simulink model "MX_Gateway"  (Simulink 9.0)
settings: Solver=FixedStepDiscrete  StartTime=0.0  StopTime=100  FixedStep=0.01  RelTol=1e-3
values from neighbouring files (symbols this model uses):
  ACTIVE = Bobcat.Parameter; CreateDefine( ACTIVE                         ,1                          ,'Define' ,'defines.h' )    [defines.m]
  ACTIVE_MODE_ONE = Bobcat.Parameter; CreateDefine( ACTIVE_MODE_ONE                ,19                         ,'Define' ,'defines.h' )    [defines.m]
  AUTHENTICATED = Bobcat.Parameter; CreateDefine( AUTHENTICATED                  ,1                          ,'Define' ,'defines.h' )    [defines.m]
  AUX_EMEA_CONTROLS = Bobcat.Parameter; CreateDefine( AUX_EMEA_CONTROLS  ,1   ,'Define' ,'defines.h' )    [enums.m]
  AUX_FLOW_HIGH = Bobcat.Parameter; CreateDefine( AUX_FLOW_HIGH           ,4  ,'Define' ,'defines.h' )    [enums.m]
  AUX_FLOW_INACTIVE = Bobcat.Parameter; CreateDefine( AUX_FLOW_INACTIVE       ,0  ,'Define' ,'defines.h' )    [enums.m]
  AUX_FLOW_LOW = Bobcat.Parameter; CreateDefine( AUX_FLOW_LOW            ,1  ,'Define' ,'defines.h' )    [enums.m]
  AUX_FLOW_MEDIUM = Bobcat.Parameter; CreateDefine( AUX_FLOW_MEDIUM         ,2  ,'Define' ,'defines.h' )    [enums.m]
  AUX_FLOW_STANDARD = Bobcat.Parameter; CreateDefine( AUX_FLOW_STANDARD       ,3  ,'Define' ,'defines.h' )    [enums.m]
  AUX_NON_FUNCTIONAL = Bobcat.Parameter; CreateDefine( AUX_NON_FUNCTIONAL ,255 ,'Define' ,'defines.h' )    [enums.m]
  BASE_ACTIVE = Bobcat.Parameter; CreateDefine( BASE_ACTIVE                    ,253                        ,'Define' ,'defines.h' )    [defines.m]
  BASE_DETENT = Bobcat.Parameter; CreateDefine( BASE_DETENT                    ,1                          ,'Define' ,'defines.h' )    [defines.m]
  BEEP_NONE = Bobcat.Parameter; CreateDefine( BEEP_NONE                      ,255                        ,'Define' ,'defines.h' )    [defines.m]
  CD_AIR_FILTER_DEBOUNCE_NORMAL = Bobcat.Parameter; CreateAliasCD( CD_AIR_FILTER_DEBOUNCE_NORMAL                        ,uint16(500)                ,'ConstantDataPointer' ,'CONSTANT_DATA' ,'AIR_FILTER_DEBOUNCE_NORMAL')    [cdata.m]    (also in hc12_cdata.m; m4_cdata.m)
  CD_AIR_FILTER_DEBOUNCE_NOT_CONNECTED = Bobcat.Parameter; CreateAliasCD( CD_AIR_FILTER_DEBOUNCE_NOT_CONNECTED                 ,uint16(3000)               ,'ConstantDataPointer' ,'CONSTANT_DATA' ,'AIR_FILTER_DEBOUNCE_NOT_CONNECTED')    [cdata.m]    (also in hc12_cdata.m; m4_cdata.m)
  CD_AIR_FILTER_DEBOUNCE_PLUGGED = Bobcat.Parameter; CreateAliasCD( CD_AIR_FILTER_DEBOUNCE_PLUGGED                       ,uint16(3000)               ,'ConstantDataPointer' ,'CONSTANT_DATA' ,'AIR_FILTER_DEBOUNCE_PLUGGED')    [cdata.m]    (also in hc12_cdata.m; m4_cdata.m)
  CD_AIR_FILTER_FUNCTION = Bobcat.Parameter; CreateAliasCD( CD_AIR_FILTER_FUNCTION                               ,uint8(255)                 ,'ConstantDataPointer' ,'CONSTANT_DATA' ,'AIR_FILTER_FUNCTION')    [cdata.m]    (also in hc12_cdata.m; m4_cdata.m)
  CD_AIR_FILTER_PLUGGED_WEIGHT = Bobcat.Parameter; CreateAliasCD( CD_AIR_FILTER_PLUGGED_WEIGHT                         ,uint8(2)                   ,'ConstantDataPointer' ,'CONSTANT_DATA' ,'AIR_FILTER_PLUGGED_WEIGHT')    [cdata.m]    (also in hc12_cdata.m; m4_cdata.m)
  CD_AIR_FILTER_UNPLUGGED_WEIGHT = Bobcat.Parameter; CreateAliasCD( CD_AIR_FILTER_UNPLUGGED_WEIGHT                       ,uint8(1)                   ,'ConstantDataPointer' ,'CONSTANT_DATA' ,'AIR_FILTER_UNPLUGGED_WEIGHT')    [cdata.m]    (also in hc12_cdata.m; m4_cdata.m)
  CD_AUTOIDLE_ARMBAR_DELAY = Bobcat.Parameter; CreateAliasCD( CD_AUTOIDLE_ARMBAR_DELAY                             ,uint8(2)                   ,'ConstantDataPointer' ,'CONSTANT_DATA' ,'AUTOIDLE_ARMBAR_DELAY')    [cdata.m]    (also in hc12_cdata.m; m4_cdata.m)
  CD_AUTOIDLE_FUNCTION = Bobcat.Parameter; CreateAliasCD( CD_AUTOIDLE_FUNCTION                                 ,uint8(255)                 ,'ConstantDataPointer' ,'CONSTANT_DATA' ,'AUTOIDLE_FUNCTION')    [cdata.m]    (also in hc12_cdata.m; m4_cdata.m)
  CD_AUTOIDLE_IDLE_DELAY = Bobcat.Parameter; CreateAliasCD( CD_AUTOIDLE_IDLE_DELAY                               ,uint8(40)                  ,'ConstantDataPointer' ,'CONSTANT_DATA' ,'AUTOIDLE_IDLE_DELAY')    [cdata.m]    (also in hc12_cdata.m; m4_cdata.m)
  CD_AUTOIDLE_LOW_IDLE_START = Bobcat.Parameter; CreateAliasCD( CD_AUTOIDLE_LOW_IDLE_START                           ,uint8(255)                 ,'ConstantDataPointer' ,'CONSTANT_DATA' ,'AUTOIDLE_LOW_IDLE_START')    [cdata.m]    (also in hc12_cdata.m; m4_cdata.m)
  CD_AUTOIDLE_PRESSURE_OFF = Bobcat.Parameter; CreateAliasCD( CD_AUTOIDLE_PRESSURE_OFF                             ,uint16(300)                ,'ConstantDataPointer' ,'CONSTANT_DATA' ,'AUTOIDLE_PRESSURE_OFF')    [cdata.m]    (also in hc12_cdata.m; m4_cdata.m)
  CD_AUTOIDLE_PRESSURE_ON = Bobcat.Parameter; CreateAliasCD( CD_AUTOIDLE_PRESSURE_ON                              ,uint16(270)                ,'ConstantDataPointer' ,'CONSTANT_DATA' ,'AUTOIDLE_PRESSURE_ON')    [cdata.m]    (also in hc12_cdata.m; m4_cdata.m)
  CD_AUTOSHIFT_FUNCTION = Bobcat.Parameter; CreateAliasCD( CD_AUTOSHIFT_FUNCTION                                ,uint8(255)                 ,'ConstantDataPointer' ,'CONSTANT_DATA' ,'AUTOSHIFT_FUNCTION')    [cdata.m]    (also in hc12_cdata.m; m4_cdata.m)
  CD_AUTOSHIFT_PHIGH1 = Bobcat.Parameter; CreateAliasCD( CD_AUTOSHIFT_PHIGH1                                  ,uint16(205)                ,'ConstantDataPointer' ,'CONSTANT_DATA' ,'AUTOSHIFT_PHIGH1')    [cdata.m]    (also in hc12_cdata.m; m4_cdata.m)
  CD_AUTOSHIFT_PHIGH2 = Bobcat.Parameter; CreateAliasCD( CD_AUTOSHIFT_PHIGH2                                  ,uint16(193)                ,'ConstantDataPointer' ,'CONSTANT_DATA' ,'AUTOSHIFT_PHIGH2')    [cdata.m]    (also in hc12_cdata.m; m4_cdata.m)
  CD_AUTOSHIFT_PHIGH_TEMP1 = Bobcat.Parameter; CreateAliasCD( CD_AUTOSHIFT_PHIGH_TEMP1                             ,int16(70)                  ,'ConstantDataPointer' ,'CONSTANT_DATA' ,'AUTOSHIFT_PHIGH_TEMP1')    [cdata.m]    (also in hc12_cdata.m; m4_cdata.m)
  CD_AUTOSHIFT_PHIGH_TEMP2 = Bobcat.Parameter; CreateAliasCD( CD_AUTOSHIFT_PHIGH_TEMP2                             ,int16(160)                 ,'ConstantDataPointer' ,'CONSTANT_DATA' ,'AUTOSHIFT_PHIGH_TEMP2')    [cdata.m]    (also in hc12_cdata.m; m4_cdata.m)
  CD_AUTOSHIFT_PLOW1 = Bobcat.Parameter; CreateAliasCD( CD_AUTOSHIFT_PLOW1                                   ,uint16(130)                ,'ConstantDataPointer' ,'CONSTANT_DATA' ,'AUTOSHIFT_PLOW1')    [cdata.m]    (also in hc12_cdata.m; m4_cdata.m)
  CD_AUTOSHIFT_PLOW2 = Bobcat.Parameter; CreateAliasCD( CD_AUTOSHIFT_PLOW2                                   ,uint16(100)                ,'ConstantDataPointer' ,'CONSTANT_DATA' ,'AUTOSHIFT_PLOW2')    [cdata.m]    (also in hc12_cdata.m; m4_cdata.m)
  CD_AUTOSHIFT_PLOW_TEMP1 = Bobcat.Parameter; CreateAliasCD( CD_AUTOSHIFT_PLOW_TEMP1                              ,int16(20)                  ,'ConstantDataPointer' ,'CONSTANT_DATA' ,'AUTOSHIFT_PLOW_TEMP1')    [cdata.m]    (also in hc12_cdata.m; m4_cdata.m)
  CD_AUTOSHIFT_PLOW_TEMP2 = Bobcat.Parameter; CreateAliasCD( CD_AUTOSHIFT_PLOW_TEMP2                              ,int16(80)                  ,'ConstantDataPointer' ,'CONSTANT_DATA' ,'AUTOSHIFT_PLOW_TEMP2')    [cdata.m]    (also in hc12_cdata.m; m4_cdata.m)
  CD_AUTOSHIFT_TRAVEL_DELAY = Bobcat.Parameter; CreateAliasCD( CD_AUTOSHIFT_TRAVEL_DELAY                            ,uint8(32)                  ,'ConstantDataPointer' ,'CONSTANT_DATA' ,'AUTOSHIFT_TRAVEL_DELAY')    [cdata.m]    (also in hc12_cdata.m; m4_cdata.m)
  CD_AUX2_CRACKPOINT_CURRENT = Bobcat.Parameter; CreateAliasCD( CD_AUX2_CRACKPOINT_CURRENT                           ,fi(0,0,16,12,'hex','0c28') ,'ConstantDataPointer' ,'CONSTANT_DATA' ,'AUX2_CRACKPOINT_CURRENT')    [cdata.m]    (also in hc12_cdata.m; m4_cdata.m)
  CD_AUX2_FLOW_LOW_FUNCTION = Bobcat.Parameter; CreateAliasCD( CD_AUX2_FLOW_LOW_FUNCTION                            ,uint8(255)                 ,'ConstantDataPointer' ,'CONSTANT_DATA' ,'AUX2_FLOW_LOW_FUNCTION')    [cdata.m]    (also in hc12_cdata.m; m4_cdata.m)
  CD_AUX2_FLOW_MED_FUNCTION = Bobcat.Parameter; CreateAliasCD( CD_AUX2_FLOW_MED_FUNCTION                            ,uint8(255)                 ,'ConstantDataPointer' ,'CONSTANT_DATA' ,'AUX2_FLOW_MED_FUNCTION')    [cdata.m]    (also in hc12_cdata.m; m4_cdata.m)
  CD_AUX2_HI_FLOW_PERCENT = Bobcat.Parameter; CreateAliasCD( CD_AUX2_HI_FLOW_PERCENT                              ,fi(0,0,16,16,'hex','ffff') ,'ConstantDataPointer' ,'CONSTANT_DATA' ,'AUX2_HI_FLOW_PERCENT')    [cdata.m]    (also in hc12_cdata.m; m4_cdata.m)
  CD_AUX2_LOW_FLOW_PERCENT = Bobcat.Parameter; CreateAliasCD( CD_AUX2_LOW_FLOW_PERCENT                             ,fi(0,0,16,16,'hex','7333') ,'ConstantDataPointer' ,'CONSTANT_DATA' ,'AUX2_LOW_FLOW_PERCENT')    [cdata.m]    (also in hc12_cdata.m; m4_cdata.m)
  CD_AUX2_MAX_FLOW_CURRENT = Bobcat.Parameter; CreateAliasCD( CD_AUX2_MAX_FLOW_CURRENT                             ,fi(0,0,16,12,'hex','14cc') ,'ConstantDataPointer' ,'CONSTANT_DATA' ,'AUX2_MAX_FLOW_CURRENT')    [cdata.m]    (also in hc12_cdata.m; m4_cdata.m)
  CD_AUX2_MED_FLOW_PERCENT = Bobcat.Parameter; CreateAliasCD( CD_AUX2_MED_FLOW_PERCENT                             ,fi(0,0,16,16,'hex','9999') ,'ConstantDataPointer' ,'CONSTANT_DATA' ,'AUX2_MED_FLOW_PERCENT')    [cdata.m]    (also in hc12_cdata.m; m4_cdata.m)
  CD_AUX_CRACKPOINT_CURRENT = Bobcat.Parameter; CreateAliasCD( CD_AUX_CRACKPOINT_CURRENT                            ,fi(0,0,16,12,'hex','0000') ,'ConstantDataPointer' ,'CONSTANT_DATA' ,'AUX_CRACKPOINT_CURRENT')    [cdata.m]    (also in hc12_cdata.m; m4_cdata.m)
  CD_AUX_DETENT_FLASH_TIME = Bobcat.Parameter; CreateAliasCD( CD_AUX_DETENT_FLASH_TIME                             ,uint16(72)                 ,'ConstantDataPointer' ,'CONSTANT_DATA' ,'AUX_DETENT_FLASH_TIME')    [cdata.m]    (also in hc12_cdata.m; m4_cdata.m)
  CD_AUX_DETENT_HOLD_TIME = Bobcat.Parameter; CreateAliasCD( CD_AUX_DETENT_HOLD_TIME                              ,uint16(100)                ,'ConstantDataPointer' ,'CONSTANT_DATA' ,'AUX_DETENT_HOLD_TIME')    [cdata.m]    (also in hc12_cdata.m; m4_cdata.m)
  CD_AUX_FULL_ON_AUX1 = Bobcat.Parameter; CreateAliasCD( CD_AUX_FULL_ON_AUX1                                  ,fi(0,0,16,12,'hex','10cd') ,'ConstantDataPointer' ,'CONSTANT_DATA' ,'AUX_FULL_ON_AUX1')    [cdata.m]    (also in hc12_cdata.m; m4_cdata.m)
  CD_AUX_FULL_ON_AUX2 = Bobcat.Parameter; CreateAliasCD( CD_AUX_FULL_ON_AUX2                                  ,fi(0,0,16,12,'hex','139d') ,'ConstantDataPointer' ,'CONSTANT_DATA' ,'AUX_FULL_ON_AUX2')    [cdata.m]    (also in hc12_cdata.m; m4_cdata.m)
  CD_AUX_FULL_ON_AUX3 = Bobcat.Parameter; CreateAliasCD( CD_AUX_FULL_ON_AUX3                                  ,fi(0,0,16,12,'hex','17d7') ,'ConstantDataPointer' ,'CONSTANT_DATA' ,'AUX_FULL_ON_AUX3')    [cdata.m]    (also in hc12_cdata.m; m4_cdata.m)
  CD_AUX_FUNCTION = Bobcat.Parameter; CreateAliasCD( CD_AUX_FUNCTION                                      ,uint8(0)                   ,'ConstantDataPointer' ,'CONSTANT_DATA' ,'AUX_FUNCTION')    [cdata.m]    (also in hc12_cdata.m; m4_cdata.m)
  CD_AUX_RATE_LIMIT_CRACKPOINT = Bobcat.Parameter; CreateAliasCD( CD_AUX_RATE_LIMIT_CRACKPOINT                         ,fi(0,0,16,12,'hex','0800') ,'ConstantDataPointer' ,'CONSTANT_DATA' ,'AUX_RATE_LIMIT_CRACKPOINT')    [cdata.m]    (also in hc12_cdata.m; m4_cdata.m)
  CD_AUX_RATE_LIMIT_CRACKPOINT_RATE = Bobcat.Parameter; CreateAliasCD( CD_AUX_RATE_LIMIT_CRACKPOINT_RATE                    ,fi(0,1,32,12,'int',102400) ,'ConstantDataPointer' ,'CONSTANT_DATA' ,'AUX_RATE_LIMIT_CRACKPOINT_RATE')    [cdata.m]    (also in hc12_cdata.m; m4_cdata.m)
  CD_AUX_RATE_LIMIT_FUNCTION = Bobcat.Parameter; CreateAliasCD( CD_AUX_RATE_LIMIT_FUNCTION                           ,uint8(255)                 ,'ConstantDataPointer' ,'CONSTANT_DATA' ,'AUX_RATE_LIMIT_FUNCTION')    [cdata.m]    (also in hc12_cdata.m; m4_cdata.m)
  CD_AUX_RATE_LIMIT_MAX_ENG_SPD = Bobcat.Parameter; CreateAliasCD( CD_AUX_RATE_LIMIT_MAX_ENG_SPD                        ,uint16(1600)               ,'ConstantDataPointer' ,'CONSTANT_DATA' ,'AUX_RATE_LIMIT_MAX_ENG_SPD')    [cdata.m]    (also in hc12_cdata.m; m4_cdata.m)
  CD_AUX_RATE_LIMIT_MIN_ENG_SPD = Bobcat.Parameter; CreateAliasCD( CD_AUX_RATE_LIMIT_MIN_ENG_SPD                        ,uint16(100)                ,'ConstantDataPointer' ,'CONSTANT_DATA' ,'AUX_RATE_LIMIT_MIN_ENG_SPD')    [cdata.m]    (also in hc12_cdata.m; m4_cdata.m)
  CD_AUX_RATE_LIMIT_NORMAL_RATE = Bobcat.Parameter; CreateAliasCD( CD_AUX_RATE_LIMIT_NORMAL_RATE                        ,fi(0,1,32,12,'int',2048)   ,'ConstantDataPointer' ,'CONSTANT_DATA' ,'AUX_RATE_LIMIT_NORMAL_RATE')    [cdata.m]    (also in hc12_cdata.m; m4_cdata.m)
  CD_AUXPOT_CAL_DEADBAND_HIGH = Bobcat.Parameter; CreateAliasCD( CD_AUXPOT_CAL_DEADBAND_HIGH                          ,uint16(574)                ,'ConstantDataPointer' ,'CONSTANT_DATA' ,'AUXPOT_CAL_DEADBAND_HIGH')    [cdata.m]    (also in hc12_cdata.m; m4_cdata.m)
  CD_AUXPOT_CAL_DEADBAND_LOW = Bobcat.Parameter; CreateAliasCD( CD_AUXPOT_CAL_DEADBAND_LOW                           ,uint16(424)                ,'ConstantDataPointer' ,'CONSTANT_DATA' ,'AUXPOT_CAL_DEADBAND_LOW')    [cdata.m]    (also in hc12_cdata.m; m4_cdata.m)
  CD_AUXPOT_CENTER_RATIO = Bobcat.Parameter; CreateAliasCD( CD_AUXPOT_CENTER_RATIO                               ,uint16(500)                ,'ConstantDataPointer' ,'CONSTANT_DATA' ,'AUXPOT_CENTER_RATIO')    [cdata.m]    (also in hc12_cdata.m; m4_cdata.m)
  CD_AUXPOT_DEADBAND = Bobcat.Parameter; CreateAliasCD( CD_AUXPOT_DEADBAND                                   ,uint8(38)                  ,'ConstantDataPointer' ,'CONSTANT_DATA' ,'AUXPOT_DEADBAND')    [cdata.m]    (also in hc12_cdata.m; m4_cdata.m)
  CD_AUXPOT_FUNCTION = Bobcat.Parameter; CreateAliasCD( CD_AUXPOT_FUNCTION                                   ,uint8(0)                   ,'ConstantDataPointer' ,'CONSTANT_DATA' ,'AUXPOT_FUNCTION')    [cdata.m]    (also in hc12_cdata.m; m4_cdata.m)
  CD_AUXPOT_RATIO_GAIN = Bobcat.Parameter; CreateAliasCD( CD_AUXPOT_RATIO_GAIN                                 ,uint16(1000)               ,'ConstantDataPointer' ,'CONSTANT_DATA' ,'AUXPOT_RATIO_GAIN')    [cdata.m]    (also in hc12_cdata.m; m4_cdata.m)
  CD_CAN_THROTTLE_FUNCTION = Bobcat.Parameter; CreateAliasCD( CD_CAN_THROTTLE_FUNCTION                             ,uint8(255)                 ,'ConstantDataPointer' ,'CONSTANT_DATA' ,'CAN_THROTTLE_FUNCTION')    [cdata.m]    (also in hc12_cdata.m; m4_cdata.m)
  CD_CAN_THROTTLE_REVERSE = Bobcat.Parameter; CreateAliasCD( CD_CAN_THROTTLE_REVERSE                              ,uint8(255)                 ,'ConstantDataPointer' ,'CONSTANT_DATA' ,'CAN_THROTTLE_REVERSE')    [cdata.m]    (also in hc12_cdata.m; m4_cdata.m)
  CD_CONSOLE_DEBOUNCE_ABSENT = Bobcat.Parameter; CreateAliasCD( CD_CONSOLE_DEBOUNCE_ABSENT                           ,uint16(12)                 ,'ConstantDataPointer' ,'CONSTANT_DATA' ,'CONSOLE_DEBOUNCE_ABSENT')    [cdata.m]    (also in hc12_cdata.m; m4_cdata.m)
  CD_CONSOLE_DEBOUNCE_OORH = Bobcat.Parameter; CreateAliasCD( CD_CONSOLE_DEBOUNCE_OORH                             ,uint16(12)                 ,'ConstantDataPointer' ,'CONSTANT_DATA' ,'CONSOLE_DEBOUNCE_OORH')    [cdata.m]    (also in hc12_cdata.m; m4_cdata.m)
  CD_CONSOLE_DEBOUNCE_OORL = Bobcat.Parameter; CreateAliasCD( CD_CONSOLE_DEBOUNCE_OORL                             ,uint16(12)                 ,'ConstantDataPointer' ,'CONSTANT_DATA' ,'CONSOLE_DEBOUNCE_OORL')    [cdata.m]    (also in hc12_cdata.m; m4_cdata.m)
  CD_CONSOLE_DEBOUNCE_PRESENT = Bobcat.Parameter; CreateAliasCD( CD_CONSOLE_DEBOUNCE_PRESENT                          ,uint16(12)                 ,'ConstantDataPointer' ,'CONSTANT_DATA' ,'CONSOLE_DEBOUNCE_PRESENT')    [cdata.m]    (also in hc12_cdata.m; m4_cdata.m)
  CD_CONSOLE_FUNCTION = Bobcat.Parameter; CreateAliasCD( CD_CONSOLE_FUNCTION                                  ,uint8(0)                   ,'ConstantDataPointer' ,'CONSTANT_DATA' ,'CONSOLE_FUNCTION')    [cdata.m]    (also in hc12_cdata.m; m4_cdata.m)
  CD_CONSOLE_OORH = Bobcat.Parameter; CreateAliasCD( CD_CONSOLE_OORH                                      ,uint16(740)                ,'ConstantDataPointer' ,'CONSTANT_DATA' ,'CONSOLE_OORH')    [cdata.m]    (also in hc12_cdata.m; m4_cdata.m)
  CD_CONSOLE_OORL = Bobcat.Parameter; CreateAliasCD( CD_CONSOLE_OORL                                      ,uint16(10)                 ,'ConstantDataPointer' ,'CONSTANT_DATA' ,'CONSOLE_OORL')    [cdata.m]    (also in hc12_cdata.m; m4_cdata.m)
  CD_CONSOLE_PRESENT_HIGH = Bobcat.Parameter; CreateAliasCD( CD_CONSOLE_PRESENT_HIGH                              ,uint16(515)                ,'ConstantDataPointer' ,'CONSTANT_DATA' ,'CONSOLE_PRESENT_HIGH')    [cdata.m]    (also in hc12_cdata.m; m4_cdata.m)
  CD_CONSOLE_PRESENT_LOW = Bobcat.Parameter; CreateAliasCD( CD_CONSOLE_PRESENT_LOW                               ,uint16(375)                ,'ConstantDataPointer' ,'CONSTANT_DATA' ,'CONSOLE_PRESENT_LOW')    [cdata.m]    (also in hc12_cdata.m; m4_cdata.m)
  CD_DELUXE_GEN5_5_FUNCTION = Bobcat.Parameter; CreateAliasCD( CD_DELUXE_GEN5_5_FUNCTION                            ,uint8(255)                 ,'ConstantDataPointer' ,'CONSTANT_DATA' ,'DELUXE_GEN5_5_FUNCTION')    [cdata.m]    (also in hc12_cdata.m; m4_cdata.m)
  CD_DELUXE_GEN5_FUNCTION = Bobcat.Parameter; CreateAliasCD( CD_DELUXE_GEN5_FUNCTION                              ,uint8(255)                 ,'ConstantDataPointer' ,'CONSTANT_DATA' ,'DELUXE_GEN5_FUNCTION')    [cdata.m]    (also in hc12_cdata.m; m4_cdata.m)
  CD_DERATE_ENGINE_SPEED_DEBOUNCE_HIGH = Bobcat.Parameter; CreateAliasCD( CD_DERATE_ENGINE_SPEED_DEBOUNCE_HIGH                 ,uint16(200)                ,'ConstantDataPointer' ,'CONSTANT_DATA' ,'DERATE_ENGINE_SPEED_DEBOUNCE_HIGH')    [cdata.m]    (also in hc12_cdata.m; m4_cdata.m)
  CD_DERATE_ENGINE_SPEED_DEBOUNCE_SHUTDOWN = Bobcat.Parameter; CreateAliasCD( CD_DERATE_ENGINE_SPEED_DEBOUNCE_SHUTDOWN             ,uint16(1000)               ,'ConstantDataPointer' ,'CONSTANT_DATA' ,'DERATE_ENGINE_SPEED_DEBOUNCE_SHUTDOWN')    [cdata.m]    (also in hc12_cdata.m; m4_cdata.m)
  CD_DERATE_ENGINE_SPEED_HIGH_OFFSET = Bobcat.Parameter; CreateAliasCD( CD_DERATE_ENGINE_SPEED_HIGH_OFFSET                   ,uint16(200)                ,'ConstantDataPointer' ,'CONSTANT_DATA' ,'DERATE_ENGINE_SPEED_HIGH_OFFSET')    [cdata.m]    (also in hc12_cdata.m; m4_cdata.m)
  CD_DERATE_METHOD = Bobcat.Parameter; CreateAliasCD( CD_DERATE_METHOD                                     ,uint8(0)                   ,'ConstantDataPointer' ,'CONSTANT_DATA' ,'DERATE_METHOD')    [cdata.m]    (also in hc12_cdata.m; m4_cdata.m)
  CD_DUMP_VALVE_FUNCTION = Bobcat.Parameter; CreateAliasCD( CD_DUMP_VALVE_FUNCTION                               ,uint8(255)                 ,'ConstantDataPointer' ,'CONSTANT_DATA' ,'DUMP_VALVE_FUNCTION')    [cdata.m]    (also in hc12_cdata.m; m4_cdata.m)
  CD_DUMP_VALVE_ON_CURRENT = Bobcat.Parameter; CreateAliasCD( CD_DUMP_VALVE_ON_CURRENT                             ,fi(0,0,16,12,'hex','ffff') ,'ConstantDataPointer' ,'CONSTANT_DATA' ,'DUMP_VALVE_ON_CURRENT')    [cdata.m]    (also in hc12_cdata.m; m4_cdata.m)
  CD_ECO_MODE_THROTTLE_REDUCTION = Bobcat.Parameter; CreateAliasCD( CD_ECO_MODE_THROTTLE_REDUCTION                       ,uint16(850)                ,'ConstantDataPointer' ,'CONSTANT_DATA' ,'ECO_MODE_THROTTLE_REDUCTION')    [cdata.m]    (also in hc12_cdata.m; m4_cdata.m)
  CD_ECT_FILTER_GAIN = Bobcat.Parameter; CreateAliasCD( CD_ECT_FILTER_GAIN                                   ,uint8(6)                   ,'ConstantDataPointer' ,'CONSTANT_DATA' ,'ECT_FILTER_GAIN')    [cdata.m]    (also in hc12_cdata.m; m4_cdata.m)
  CD_ECU_FUNCTION = Bobcat.Parameter; CreateAliasCD( CD_ECU_FUNCTION                                      ,uint8(255)                 ,'ConstantDataPointer' ,'CONSTANT_DATA' ,'ECU_FUNCTION')    [cdata.m]    (also in hc12_cdata.m; m4_cdata.m)
  CD_ECU_HOLD_DEBOUNCE_ERROR_ON = Bobcat.Parameter; CreateAliasCD( CD_ECU_HOLD_DEBOUNCE_ERROR_ON                        ,uint16(20)                 ,'ConstantDataPointer' ,'CONSTANT_DATA' ,'ECU_HOLD_DEBOUNCE_ERROR_ON')    [cdata.m]    (also in hc12_cdata.m; m4_cdata.m)
  CD_EMEA_CONTROLS_HOLD_TIME = Bobcat.Parameter; CreateAliasCD( CD_EMEA_CONTROLS_HOLD_TIME                           ,uint16(30)                 ,'ConstantDataPointer' ,'CONSTANT_DATA' ,'EMEA_CONTROLS_HOLD_TIME')    [cdata.m]    (also in hc12_cdata.m; m4_cdata.m)
  CD_EMEA_CONTROLS_OPTION_A = Bobcat.Parameter; CreateAliasCD( CD_EMEA_CONTROLS_OPTION_A                            ,uint8(255)                 ,'ConstantDataPointer' ,'CONSTANT_DATA' ,'EMEA_CONTROLS_OPTION_A')    [cdata.m]    (also in hc12_cdata.m; m4_cdata.m)
  CD_EMEA_CONTROLS_OPTION_C = Bobcat.Parameter; CreateAliasCD( CD_EMEA_CONTROLS_OPTION_C                            ,uint8(255)                 ,'ConstantDataPointer' ,'CONSTANT_DATA' ,'EMEA_CONTROLS_OPTION_C')    [cdata.m]    (also in hc12_cdata.m; m4_cdata.m)
  CD_EMEA_CONTROLS_REVERSE = Bobcat.Parameter; CreateAliasCD( CD_EMEA_CONTROLS_REVERSE                             ,uint8(255)                 ,'ConstantDataPointer' ,'CONSTANT_DATA' ,'EMEA_CONTROLS_REVERSE')    [cdata.m]    (also in hc12_cdata.m; m4_cdata.m)
  CD_EMEA_ELECTRIC_VERSION = Bobcat.Parameter; CreateAliasCD( CD_EMEA_ELECTRIC_VERSION                             ,uint8(0)                   ,'ConstantDataPointer' ,'CONSTANT_DATA' ,'EMEA_ELECTRIC_VERSION')    [cdata.m]    (also in hc12_cdata.m; m4_cdata.m)
  CD_EMEA_POT_CAL_DEADBAND_HIGH = Bobcat.Parameter; CreateAliasCD( CD_EMEA_POT_CAL_DEADBAND_HIGH                        ,uint16(574)                ,'ConstantDataPointer' ,'CONSTANT_DATA' ,'EMEA_POT_CAL_DEADBAND_HIGH')    [cdata.m]    (also in hc12_cdata.m; m4_cdata.m)
  CD_EMEA_POT_CAL_DEADBAND_LOW = Bobcat.Parameter; CreateAliasCD( CD_EMEA_POT_CAL_DEADBAND_LOW                         ,uint16(424)                ,'ConstantDataPointer' ,'CONSTANT_DATA' ,'EMEA_POT_CAL_DEADBAND_LOW')    [cdata.m]    (also in hc12_cdata.m; m4_cdata.m)
  CD_ENGINE_RPM_FACTOR = Bobcat.Parameter; CreateAliasCD( CD_ENGINE_RPM_FACTOR                                 ,uint8(35)                  ,'ConstantDataPointer' ,'CONSTANT_DATA' ,'ENGINE_RPM_FACTOR')    [cdata.m]    (also in hc12_cdata.m; m4_cdata.m)
  CD_ENGINE_SPEED_FILTER_FAST_VALUE = Bobcat.Parameter; CreateAliasCD( CD_ENGINE_SPEED_FILTER_FAST_VALUE                    ,uint8(1)                   ,'ConstantDataPointer' ,'CONSTANT_DATA' ,'ENGINE_SPEED_FILTER_FAST_VALUE')    [cdata.m]    (also in hc12_cdata.m; m4_cdata.m)
  CD_ENGINE_SPEED_FILTER_SLOW_VALUE = Bobcat.Parameter; CreateAliasCD( CD_ENGINE_SPEED_FILTER_SLOW_VALUE                    ,uint8(4)                   ,'ConstantDataPointer' ,'CONSTANT_DATA' ,'ENGINE_SPEED_FILTER_SLOW_VALUE')    [cdata.m]    (also in hc12_cdata.m; m4_cdata.m)
  CD_ENGINE_SPEED_RUNNING = Bobcat.Parameter; CreateAliasCD( CD_ENGINE_SPEED_RUNNING                              ,uint16(800)                ,'ConstantDataPointer' ,'CONSTANT_DATA' ,'ENGINE_SPEED_RUNNING')    [cdata.m]    (also in hc12_cdata.m; m4_cdata.m)
  CD_ENGINE_START_DELAY_DEBOUNCE_RPM_NO_SIGNAL = Bobcat.Parameter; CreateAliasCD( CD_ENGINE_START_DELAY_DEBOUNCE_RPM_NO_SIGNAL         ,uint16(0)                  ,'ConstantDataPointer' ,'CONSTANT_DATA' ,'ENGINE_START_DELAY_DEBOUNCE_RPM_NO_SIGNAL')    [cdata.m]    (also in hc12_cdata.m; m4_cdata.m)
  CD_ENGINE_START_DELAY_OFF_TIME = Bobcat.Parameter; CreateAliasCD( CD_ENGINE_START_DELAY_OFF_TIME                       ,uint16(0)                  ,'ConstantDataPointer' ,'CONSTANT_DATA' ,'ENGINE_START_DELAY_OFF_TIME')    [cdata.m]    (also in hc12_cdata.m; m4_cdata.m)
  CD_ENGINE_START_DELAY_RPM_NO_SIGNAL = Bobcat.Parameter; CreateAliasCD( CD_ENGINE_START_DELAY_RPM_NO_SIGNAL                  ,uint16(0)                  ,'ConstantDataPointer' ,'CONSTANT_DATA' ,'ENGINE_START_DELAY_RPM_NO_SIGNAL')    [cdata.m]    (also in hc12_cdata.m; m4_cdata.m)
  CD_EOP_DEBOUNCE_EXTREMELY_LOW = Bobcat.Parameter; CreateAliasCD( CD_EOP_DEBOUNCE_EXTREMELY_LOW                        ,uint16(50)                 ,'ConstantDataPointer' ,'CONSTANT_DATA' ,'EOP_DEBOUNCE_EXTREMELY_LOW')    [cdata.m]    (also in hc12_cdata.m; m4_cdata.m)
  CD_EOP_DEBOUNCE_NORMAL = Bobcat.Parameter; CreateAliasCD( CD_EOP_DEBOUNCE_NORMAL                               ,uint16(50)                 ,'ConstantDataPointer' ,'CONSTANT_DATA' ,'EOP_DEBOUNCE_NORMAL')    [cdata.m]    (also in hc12_cdata.m; m4_cdata.m)
  CD_EOP_DEBOUNCE_SHUTDOWN = Bobcat.Parameter; CreateAliasCD( CD_EOP_DEBOUNCE_SHUTDOWN                             ,uint16(100)                ,'ConstantDataPointer' ,'CONSTANT_DATA' ,'EOP_DEBOUNCE_SHUTDOWN')    [cdata.m]    (also in hc12_cdata.m; m4_cdata.m)
  CD_EOP_FILTER_VALUE = Bobcat.Parameter; CreateAliasCD( CD_EOP_FILTER_VALUE                                  ,uint8(3)                   ,'ConstantDataPointer' ,'CONSTANT_DATA' ,'EOP_FILTER_VALUE')    [cdata.m]    (also in hc12_cdata.m; m4_cdata.m)
  CD_EOP_FUNCTION = Bobcat.Parameter; CreateAliasCD( CD_EOP_FUNCTION                                      ,uint8(1)                   ,'ConstantDataPointer' ,'CONSTANT_DATA' ,'EOP_FUNCTION')    [cdata.m]    (also in hc12_cdata.m; m4_cdata.m)
  CD_EOP_SWITCH_GAUGE_VALUE = Bobcat.Parameter; CreateAliasCD( CD_EOP_SWITCH_GAUGE_VALUE                            ,uint16(125)                ,'ConstantDataPointer' ,'CONSTANT_DATA' ,'EOP_SWITCH_GAUGE_VALUE')    [cdata.m]    (also in hc12_cdata.m; m4_cdata.m)
  CD_EOP_SWITCH_LOW = Bobcat.Parameter; CreateAliasCD( CD_EOP_SWITCH_LOW                                    ,uint16(50)                 ,'ConstantDataPointer' ,'CONSTANT_DATA' ,'EOP_SWITCH_LOW')    [cdata.m]    (also in hc12_cdata.m; m4_cdata.m)
  CD_EOP_SWITCH_PRESSURE_VALUE = Bobcat.Parameter; CreateAliasCD( CD_EOP_SWITCH_PRESSURE_VALUE                         ,uint16(40)                 ,'ConstantDataPointer' ,'CONSTANT_DATA' ,'EOP_SWITCH_PRESSURE_VALUE')    [cdata.m]    (also in hc12_cdata.m; m4_cdata.m)
  CD_EOT_DEBOUNCE_EXTREMELY_LOW = Bobcat.Parameter; CreateAliasCD( CD_EOT_DEBOUNCE_EXTREMELY_LOW                        ,uint16(2)                  ,'ConstantDataPointer' ,'CONSTANT_DATA' ,'EOT_DEBOUNCE_EXTREMELY_LOW')    [cdata.m]    (also in hc12_cdata.m; m4_cdata.m)
  CD_EOT_DEBOUNCE_LOW = Bobcat.Parameter; CreateAliasCD( CD_EOT_DEBOUNCE_LOW                                  ,uint16(2)                  ,'ConstantDataPointer' ,'CONSTANT_DATA' ,'EOT_DEBOUNCE_LOW')    [cdata.m]    (also in hc12_cdata.m; m4_cdata.m)
  CD_EOT_DEBOUNCE_NORMAL = Bobcat.Parameter; CreateAliasCD( CD_EOT_DEBOUNCE_NORMAL                               ,uint16(2)                  ,'ConstantDataPointer' ,'CONSTANT_DATA' ,'EOT_DEBOUNCE_NORMAL')    [cdata.m]    (also in hc12_cdata.m; m4_cdata.m)
  CD_EOT_EXTREMELY_LOW = Bobcat.Parameter; CreateAliasCD( CD_EOT_EXTREMELY_LOW                                 ,uint16(81)                 ,'ConstantDataPointer' ,'CONSTANT_DATA' ,'EOT_EXTREMELY_LOW')    [cdata.m]    (also in hc12_cdata.m; m4_cdata.m)
  CD_EOT_FUNCTION = Bobcat.Parameter; CreateAliasCD( CD_EOT_FUNCTION                                      ,uint8(255)                 ,'ConstantDataPointer' ,'CONSTANT_DATA' ,'EOT_FUNCTION')    [cdata.m]    (also in hc12_cdata.m; m4_cdata.m)
  CD_EOT_LOW = Bobcat.Parameter; CreateAliasCD( CD_EOT_LOW                                           ,uint16(90)                 ,'ConstantDataPointer' ,'CONSTANT_DATA' ,'EOT_LOW')    [cdata.m]    (also in hc12_cdata.m; m4_cdata.m)
  CD_EOT_TEMPERATURE_HYSTERESIS = Bobcat.Parameter; CreateAliasCD( CD_EOT_TEMPERATURE_HYSTERESIS                        ,uint16(2)                  ,'ConstantDataPointer' ,'CONSTANT_DATA' ,'EOT_TEMPERATURE_HYSTERESIS')    [cdata.m]    (also in hc12_cdata.m; m4_cdata.m)
  CD_FAST_TRAVEL_FUNCTION = Bobcat.Parameter; CreateAliasCD( CD_FAST_TRAVEL_FUNCTION                              ,uint8(255)                 ,'ConstantDataPointer' ,'CONSTANT_DATA' ,'FAST_TRAVEL_FUNCTION')    [cdata.m]    (also in hc12_cdata.m; m4_cdata.m)
  CD_FAST_TRAVEL_THROTTLE_DELAY = Bobcat.Parameter; CreateAliasCD( CD_FAST_TRAVEL_THROTTLE_DELAY                        ,uint16(10)                 ,'ConstantDataPointer' ,'CONSTANT_DATA' ,'FAST_TRAVEL_THROTTLE_DELAY')    [cdata.m]    (also in hc12_cdata.m; m4_cdata.m)
  CD_FAST_TRAVEL_THROTTLE_LIMIT = Bobcat.Parameter; CreateAliasCD( CD_FAST_TRAVEL_THROTTLE_LIMIT                        ,uint16(1000)               ,'ConstantDataPointer' ,'CONSTANT_DATA' ,'FAST_TRAVEL_THROTTLE_LIMIT')    [cdata.m]    (also in hc12_cdata.m; m4_cdata.m)
  CD_FLYWHEEL_TEETH = Bobcat.Parameter; CreateAliasCD( CD_FLYWHEEL_TEETH                                    ,uint8(83)                  ,'ConstantDataPointer' ,'CONSTANT_DATA' ,'FLYWHEEL_TEETH')    [cdata.m]    (also in hc12_cdata.m; m4_cdata.m)
  CD_FUEL_LEVEL_FILTER_FAST_VALUE = Bobcat.Parameter; CreateAliasCD( CD_FUEL_LEVEL_FILTER_FAST_VALUE                      ,uint8(5)                   ,'ConstantDataPointer' ,'CONSTANT_DATA' ,'FUEL_LEVEL_FILTER_FAST_VALUE')    [cdata.m]    (also in hc12_cdata.m; m4_cdata.m)
  CD_FUEL_LEVEL_FILTER_SLOW_VALUE = Bobcat.Parameter; CreateAliasCD( CD_FUEL_LEVEL_FILTER_SLOW_VALUE                      ,uint8(10)                  ,'ConstantDataPointer' ,'CONSTANT_DATA' ,'FUEL_LEVEL_FILTER_SLOW_VALUE')    [cdata.m]    (also in hc12_cdata.m; m4_cdata.m)
  CD_FUEL_PRIMARY_FEEDBACK_FUNCTION = Bobcat.Parameter; CreateAliasCD( CD_FUEL_PRIMARY_FEEDBACK_FUNCTION                    ,uint8(0)                   ,'ConstantDataPointer' ,'CONSTANT_DATA' ,'FUEL_PRIMARY_FEEDBACK_FUNCTION')    [cdata.m]    (also in hc12_cdata.m; m4_cdata.m)
  ... (+246 more)
undefined symbols (not defined in the file or in its folder): sigbuilder_block
undefined symbols (not defined in the file): CD_FUEL_RESOLUTION_TIMER
libraries: simulink x186, MX_Library x107
(part 32 of 48)

== stateflow chart MX_Gtwy_Control/System/Aux_Enable/Aux_Control ==
  state Detent_State [parallel]
    settings: executionOrder=2
    state Non_Functional
      en:
      state_DetentEnable = INACTIVE;
      state_Detent = NO_DETENT;
    state EMEA_CONTROLS
      state Enable [parallel]
        settings: executionOrder=1
        state Disabled
          en:
          state_DetentEnable = INACTIVE;
        state Enabled
          en:
          state_DetentEnable = ACTIVE;
        (default) -> Detent_State.EMEA_CONTROLS.Enable.Disabled
        Detent_State.EMEA_CONTROLS.Enable.Disabled -> Detent_State.EMEA_CONTROLS.Enable.Enabled : [state_AuxEnable == ACTIVE_MODE_ONE...
            | && aux_DetentHold == ACTIVE...
            | && state_Buttons == NORMAL...
            | && mode_RightControl ==MODE_RIGHT_AUX1]
            | {state_Buzzer = SINGLE_SHORT_BEEP;}
        Detent_State.EMEA_CONTROLS.Enable.Enabled -> Detent_State.EMEA_CONTROLS.Enable.Disabled [order 1] : [state_Buttons != NORMAL...
            | || state_AuxEnable == INACTIVE...
            | || state_Console != ACTIVE]
        Detent_State.EMEA_CONTROLS.Enable.Enabled -> Detent_State.EMEA_CONTROLS.Enable.Disabled [order 2] : [aux_DetentHold == ACTIVE]
            | {send(disable_aux,Aux_Enable_State);
            | state_Buzzer = SINGLE_SHORT_BEEP;}
        Detent_State.EMEA_CONTROLS.Enable.Enabled -> Detent_State.EMEA_CONTROLS.Enable.Disabled [order 3] : disable_detent
        Detent_State.EMEA_CONTROLS.Enable.Enabled -> Detent_State.EMEA_CONTROLS.Enable.Disabled [order 4] : [mode_RightControl != MODE_RIGHT_AUX1]
      state Detent [parallel]
        settings: executionOrder=2
        state Active_Normal
          state Detent_Base
            en:
            state_Detent = BASE_DETENT;
          state Detent_Rod
            en:
            state_Detent = ROD_DETENT;
          (junction 2081) -> Detent_State.EMEA_CONTROLS.Detent.Active_Normal.Detent_Base [order 2]
          Detent_State.EMEA_CONTROLS.Detent.Active_Normal.Detent_Rod -> Detent_State.EMEA_CONTROLS.Detent.Active_Normal.Detent_Base [order 1] : [DetentRisingEdge &&...
              |  state_AuxPot ==...
              |  BASE_ACTIVE]
          Detent_State.EMEA_CONTROLS.Detent.Active_Normal.Detent_Base -> Detent_State.EMEA_CONTROLS.Detent.Active_Normal.Detent_Rod [order 1] : [DetentRisingEdge &&...
              |  state_RightPot ==...
              |  ROD_ACTIVE]
          (junction 2081) -> Detent_State.EMEA_CONTROLS.Detent.Active_Normal.Detent_Rod [order 1] : [state_RightPot ==...
              |  ROD_ACTIVE]
        state No_Detent
          en:
          state_Detent = NO_DETENT;
        state Not_Enabled
          en:
          state_Detent = ERR_DETENT;
          state_Buzzer = DOUBLE_SHORT_BEEP;
        (default) -> Detent_State.EMEA_CONTROLS.Detent.No_Detent
        Detent_State.EMEA_CONTROLS.Detent.Active_Normal.Detent_Base -> Detent_State.EMEA_CONTROLS.Detent.No_Detent [order 2] : [DetentRisingEdge]
        Detent_State.EMEA_CONTROLS.Detent.No_Detent -> Detent_State.EMEA_CONTROLS.Detent.Not_Enabled [order 2] : [DetentRisingEdge...
            |  && mode_RightControl ==MODE_RIGHT_AUX1...
            |  && state_DetentEnable == INACTIVE...
            |  && state_AuxEnable == ACTIVE_MODE_ONE...
            |  && state_RightPot != NOT_IN_NEUTRAL]
        Detent_State.EMEA_CONTROLS.Detent.Not_Enabled -> Detent_State.EMEA_CONTROLS.Detent.No_Detent : [after(CD_AUX_DETENT_FLASH_TIME * 10, tick)]
        Detent_State.EMEA_CONTROLS.Detent.No_Detent -> (junction 2081) [order 1] : [DetentRisingEdge...
            |  && mode_RightControl ==MODE_RIGHT_AUX1...
            |  && state_DetentEnable == ACTIVE...
            |  && state_AuxEnable == ACTIVE_MODE_ONE...
            |  && state_RightPot != NOT_IN_NEUTRAL ]
        Detent_State.EMEA_CONTROLS.Detent.Active_Normal.Detent_Rod -> Detent_State.EMEA_CONTROLS.Detent.No_Detent [order 2] : [DetentRisingEdge]
        Detent_State.EMEA_CONTROLS.Detent.Active_Normal -> Detent_State.EMEA_CONTROLS.Detent.No_Detent : [aux_DebounceButton...
            | || state_DetentEnable == INACTIVE...
            | || state_AuxEnable == INACTIVE]
    state NORMAL_CONTROLS
      state Enable [parallel]
        settings: executionOrder=1
        state Disabled
          en:
          state_DetentEnable = INACTIVE;
        state Enabled
          en:
          state_DetentEnable = ACTIVE;
        (default) -> Detent_State.NORMAL_CONTROLS.Enable.Disabled
        Detent_State.NORMAL_CONTROLS.Enable.Disabled -> Detent_State.NORMAL_CONTROLS.Enable.Enabled : [state_AuxEnable == ACTIVE_MODE_ONE...
            | && aux_DetentHold == ACTIVE...
            | && state_Buttons == NORMAL]
        Detent_State.NORMAL_CONTROLS.Enable.Enabled -> Detent_State.NORMAL_CONTROLS.Enable.Disabled [order 1] : [state_Buttons != NORMAL...
            | || state_AuxEnable == INACTIVE...
            | || state_Console != ACTIVE]
        Detent_State.NORMAL_CONTROLS.Enable.Enabled -> Detent_State.NORMAL_CONTROLS.Enable.Disabled [order 2] : [aux_DetentHold == ACTIVE]
            | {send(disable_aux,Aux_Enable_State);}
        Detent_State.NORMAL_CONTROLS.Enable.Enabled -> Detent_State.NORMAL_CONTROLS.Enable.Disabled [order 3] : disable_detent
      state Detent [parallel]
        settings: executionOrder=2
        state Active_Normal
          state Detent_Base
            en:
            state_Detent = BASE_DETENT;
          state Detent_Rod
            en:
            state_Detent = ROD_DETENT;
          (junction 2098) -> Detent_State.NORMAL_CONTROLS.Detent.Active_Normal.Detent_Base [order 2]
          Detent_State.NORMAL_CONTROLS.Detent.Active_Normal.Detent_Rod -> Detent_State.NORMAL_CONTROLS.Detent.Active_Normal.Detent_Base [order 1] : [DetentRisingEdge &&...
              |  state_AuxPot ==...
              |  BASE_ACTIVE]
          Detent_State.NORMAL_CONTROLS.Detent.Active_Normal.Detent_Base -> Detent_State.NORMAL_CONTROLS.Detent.Active_Normal.Detent_Rod [order 1] : [DetentRisingEdge &&...
              |  state_AuxPot ==...
              |  ROD_ACTIVE]
          (junction 2098) -> Detent_State.NORMAL_CONTROLS.Detent.Active_Normal.Detent_Rod [order 1] : [state_AuxPot ==...
              |  ROD_ACTIVE]
        state No_Detent
          en:
          state_Detent = NO_DETENT;
        state Not_Enabled
          en:
          state_Detent = ERR_DETENT;
          state_Buzzer = DOUBLE_SHORT_BEEP;
        (default) -> Detent_State.NORMAL_CONTROLS.Detent.No_Detent
        Detent_State.NORMAL_CONTROLS.Detent.Active_Normal.Detent_Base -> Detent_State.NORMAL_CONTROLS.Detent.No_Detent [order 2] : [DetentRisingEdge]
        Detent_State.NORMAL_CONTROLS.Detent.No_Detent -> Detent_State.NORMAL_CONTROLS.Detent.Not_Enabled [order 2] : [DetentRisingEdge...
            |  && state_DetentEnable == INACTIVE...
            |  && state_AuxEnable == ACTIVE_MODE_ONE...
            |  && state_AuxPot != NOT_IN_NEUTRAL]
        Detent_State.NORMAL_CONTROLS.Detent.No_Detent -> (junction 2098) [order 1] : [DetentRisingEdge...
            |  && state_DetentEnable == ACTIVE...
            |  && state_AuxEnable == ACTIVE_MODE_ONE...
            |  && state_AuxPot != NOT_IN_NEUTRAL ]
        Detent_State.NORMAL_CONTROLS.Detent.Not_Enabled -> Detent_State.NORMAL_CONTROLS.Detent.No_Detent : [after(CD_AUX_DETENT_FLASH_TIME * 10, tick)]
        Detent_State.NORMAL_CONTROLS.Detent.Active_Normal.Detent_Rod -> Detent_State.NORMAL_CONTROLS.Detent.No_Detent [order 2] : [DetentRisingEdge]
        Detent_State.NORMAL_CONTROLS.Detent.Active_Normal -> Detent_State.NORMAL_CONTROLS.Detent.No_Detent : [aux_DebounceButton...
            | || state_DetentEnable == INACTIVE...
            | || state_AuxEnable == INACTIVE]
    (default) -> Detent_State.Non_Functional
    Detent_State.Non_Functional -> (junction 2063)
    (junction 2063) -> Detent_State.EMEA_CONTROLS [order 1] : [CD_RIGHT_POT_FUNCTION !=NON_FUNCTIONAL]
    (junction 2063) -> NORMAL_CONTROLS [order 2] : [CD_AUX_FUNCTION != NON_FUNCTIONAL]
  state Aux_Enable_State [parallel]
    settings: executionOrder=1
    state Non_Functional
      en:
      state_AuxEnable = INACTIVE;
      aux_Flow = AUX_FLOW_INACTIVE;
    state PHOENIX_K2
      state Functional
        state Enabled
          state Off
            en:
            aux_Flow = AUX_FLOW_INACTIVE;
            ee_AuxFlow = AUX_FLOW_INACTIVE;
            state_AuxEnable = INACTIVE;
            matlab_UpdateEEVar();
          state Active
            state Low_Flow
              en:
              aux_Flow = AUX_FLOW_LOW;
              ee_AuxFlow = AUX_FLOW_LOW;
              state_AuxEnable = ACTIVE_MODE_ONE;
              matlab_UpdateEEVar();
            state Medium_Flow
              en:
              aux_Flow = AUX_FLOW_MEDIUM;
              ee_AuxFlow = AUX_FLOW_MEDIUM;
              state_AuxEnable = ACTIVE_MODE_ONE;
              matlab_UpdateEEVar();
            state Standard_Flow
              en:
              aux_Flow = AUX_FLOW_STANDARD;
              ee_AuxFlow = AUX_FLOW_STANDARD;
              state_AuxEnable = ACTIVE_MODE_ONE;
              matlab_UpdateEEVar();
            Aux_Enable_State.PHOENIX_K2.Functional.Enabled.Active.Medium_Flow -> Aux_Enable_State.PHOENIX_K2.Functional.Enabled.Active.Low_Flow : [aux_DebounceButton]
                | {state_Buzzer = SINGLE_SHORT_BEEP;}
            Aux_Enable_State.PHOENIX_K2.Functional.Enabled.Active.Low_Flow -> Aux_Enable_State.PHOENIX_K2.Functional.Enabled.Active.Standard_Flow [order 1] : [aux_DebounceButton...
                | && state_DetentEnable == ACTIVE]
                | {state_Buzzer = SINGLE_SHORT_BEEP;}
            (junction 2153) -> (junction 2154) [order 1] : [CD_AUX_ADJ_FLOW_FUNCTION == NON_FUNCTIONAL]
            (junction 2153) -> Aux_Enable_State.PHOENIX_K2.Functional.Enabled.Active.Standard_Flow [order 2] : {state_Buzzer = SINGLE_SHORT_BEEP;}
            (junction 2154) -> Aux_Enable_State.PHOENIX_K2.Functional.Enabled.Active.Standard_Flow
            Aux_Enable_State.PHOENIX_K2.Functional.Enabled.Active.Standard_Flow -> Aux_Enable_State.PHOENIX_K2.Functional.Enabled.Active.Medium_Flow [order 1] : [aux_DebounceButton...
                | && CD_AUX_ADJ_FLOW_FUNCTION != NON_FUNCTIONAL]
                | {state_Buzzer = SINGLE_SHORT_BEEP;}
          (default) -> (junction 2137)
          (junction 2137) -> Aux_Enable_State.PHOENIX_K2.Functional.Enabled.Off [order 1] : [ee_AuxFlow == AUX_FLOW_INACTIVE]
          (junction 2137) -> (junction 2138) [order 2]
          Aux_Enable_State.PHOENIX_K2.Functional.Enabled.Active.Low_Flow -> Aux_Enable_State.PHOENIX_K2.Functional.Enabled.Off [order 2] : [aux_DebounceButton...
              | && state_DetentEnable != ACTIVE]
              | {state_Buzzer = SINGLE_SHORT_BEEP;}
          Aux_Enable_State.PHOENIX_K2.Functional.Enabled.Off -> (junction 2153) : [aux_DebounceButton...
              | || aux_DetentHold]
          Aux_Enable_State.PHOENIX_K2.Functional.Enabled.Active.Standard_Flow -> Aux_Enable_State.PHOENIX_K2.Functional.Enabled.Off [order 2] : [aux_DebounceButton...
              | && CD_AUX_ADJ_FLOW_FUNCTION == NON_FUNCTIONAL]
          Aux_Enable_State.PHOENIX_K2.Functional.Enabled.Active -> Aux_Enable_State.PHOENIX_K2.Functional.Enabled.Off : disable_aux
          (junction 2138) -> Aux_Enable_State.PHOENIX_K2.Functional.Enabled.Active.Low_Flow [order 1] : [ee_AuxFlow == AUX_FLOW_LOW...
              | && CD_AUX_ADJ_FLOW_FUNCTION != NON_FUNCTIONAL]
          (junction 2138) -> (junction 2139) [order 2]
          (junction 2139) -> Aux_Enable_State.PHOENIX_K2.Functional.Enabled.Active.Medium_Flow [order 1] : [ee_AuxFlow == AUX_FLOW_MEDIUM...
              | && CD_AUX_ADJ_FLOW_FUNCTION != NON_FUNCTIONAL]
          (junction 2139) -> (junction 2140) [order 2]
          (junction 2140) -> Aux_Enable_State.PHOENIX_K2.Functional.Enabled.Active.Standard_Flow
        state Inactive
          en:
          state_AuxEnable = INACTIVE;
          cmd_AuxRelease = INACTIVE;
          aux_Flow = AUX_FLOW_INACTIVE;
          state_AuxRelease = INACTIVE;
        state Inactive_Fault
          en:
          state_AuxEnable = INACTIVE;
          aux_Flow = AUX_FLOW_INACTIVE;
        state Inactive_Out_Of_Neutral
          en:
          state_AuxEnable = INACTIVE;
          aux_Flow = AUX_FLOW_INACTIVE;
        state Aux_Relief_G5